simulink model "fftw_15_2_18_18"  (Simulink 7.9)
settings: Solver=ode45  StartTime=0.0  StopTime=10.0  RelTol=1e-3
libraries: xbsIndex_r4 x2224, casper_library_misc x104, casper_library_delays x17, xbsReplacements_r4 x2, casper_library_ffts_twiddle x1, casper_library_reorder x1
(part 19 of 37)

== system fftw_15_2_18_18/fft_wideband_real/fft_direct/butterfly2_1/twiddle_general_4mult/coeff_gen ==
  rst : Inport
  Counter : xbsIndex_r4/Counter  cnt_type=Free Running  cnt_to=Inf  operation=Up  start_count=0  cnt_by_val=1  arith_type=Unsigned  n_bits=13  bin_pt=0  load_pin=off  rst=on  en=off  explicit_period=on  period=1  dbl_ovrd=off  use_behavioral_HDL=off  implementation=Fabric  block_type=counter
  ROM : xbsIndex_r4/ROM  depth=8192  initVector="[0                    -0.0001983642578125                    -0.0003814697265625                     -0.000579833984375                     -0.000762939453125                    -0.0009613037109375                    -0.0011444091796875                       -0.0013427734375                    -0.0015411376953125                    -0.0017242431640625                     -0.001922607421875                     -0.002105712890625                    -0.0023040771484375                    -0.0024871826171875                        -0.002685546875                      -0.00286865234375                    -0.0030670166015625                     -0.003265380859375                     -0.003448486328125                    -0.0036468505859375                    -0.0038299560546875                       -0.0040283203125                      -0.00421142578125                    -0.0044097900390625                     -0.004608154296875                     -0.004791259765625                    -0.0049896240234375                    -0.0051727294921875                         -0.00537109375                      -0.00555419921875                    -0.0057525634765625                     -0.005950927734375                     -0.006134033203125                    -0.0063323974609375                    -0.0065155029296875                       -0.0067138671875                      -0.00689697265625                    -0.0070953369140625                     -0.007293701171875                     -0.007476806640625                    -0.0076751708984375                    -0.0078582763671875                        -0.008056640625                      -0.00823974609375                    -0.0084381103515625                    -0.0086212158203125                     -0.008819580078125                    -0.0090179443359375                    -0.0092010498046875                       -0.0093994140625                      -0.00958251953125                    -0.0097808837890625                    -0.0099639892578125                     -0.010162353515625                    -0.0103607177734375                    -0.0105438232421875                          -0.0107421875                      -0.01092529296875                    -0.0111236572265625                    -0.0113067626953125                     -0.011505126953125                    -0.0117034912109375                    -0.0118865966796875                       -0.0120849609375                      -0.01226806640625                    -0.0124664306640625                    -0.0126495361328125                     -0.012847900390625                     -0.013031005859375                    -0.0132293701171875                        -0.013427734375                      -0.01361083984375                    -0.0138092041015625                    -0.0139923095703125                     -0.014190673828125                     -0.014373779296875                    -0.0145721435546875                       -0.0147705078125                      -0.01495361328125                    -0.0151519775390625                    -0.0153350830078125                     -0.015533447265625                     -0.015716552734375                    -0.0159149169921875                         -0.01611328125                      -0.01629638671875                    -0.0164947509765625                    -0.0166778564453125                     -0.016876220703125                     -0.017059326171875                    -0.0172576904296875                    -0.0174407958984375                      -0.01763916015625                    -0.0178375244140625                    -0.0180206298828125                     -0.018218994140625                     -0.018402099609375                    -0.0186004638671875                    -0.0187835693359375                      -0.01898193359375                    -0.0191802978515625                    -0.0193634033203125                     -0.019561767578125 ...(+315452 chars)]"  distributed_mem=Block RAM  rst=off  init_reg=0  en=off  latency=2  gui_display_data_type=Fixed-point  arith_type="Signed  (2's comp)"  n_bits=18  bin_pt=16  preci_type=Single  exp_width=8  frac_width=24  dbl_ovrd=off  optimize=Area  use_rpm=on  block_type=sprom
  ROM1 : xbsIndex_r4/ROM  depth=8192  initVector="[-1                                     -1                                     -1                                     -1                                     -1                                     -1                                     -1                                     -1                                     -1                                     -1                                     -1                                     -1                                     -1                                     -1                                     -1                                     -1                                     -1                                     -1                                     -1                                     -1                                     -1                    -0.9999847412109375                    -0.9999847412109375                    -0.9999847412109375                    -0.9999847412109375                    -0.9999847412109375                    -0.9999847412109375                    -0.9999847412109375                    -0.9999847412109375                    -0.9999847412109375                    -0.9999847412109375                    -0.9999847412109375                    -0.9999847412109375                    -0.9999847412109375                    -0.9999847412109375                    -0.9999847412109375                     -0.999969482421875                     -0.999969482421875                     -0.999969482421875                     -0.999969482421875                     -0.999969482421875                     -0.999969482421875                     -0.999969482421875                     -0.999969482421875                     -0.999969482421875                     -0.999969482421875                    -0.9999542236328125                    -0.9999542236328125                    -0.9999542236328125                    -0.9999542236328125                    -0.9999542236328125                    -0.9999542236328125                    -0.9999542236328125                    -0.9999542236328125                      -0.99993896484375                      -0.99993896484375                      -0.99993896484375                      -0.99993896484375                      -0.99993896484375                      -0.99993896484375                      -0.99993896484375                      -0.99993896484375                    -0.9999237060546875                    -0.9999237060546875                    -0.9999237060546875                    -0.9999237060546875                    -0.9999237060546875                    -0.9999237060546875                     -0.999908447265625                     -0.999908447265625                     -0.999908447265625                     -0.999908447265625                     -0.999908447265625                     -0.999908447265625                    -0.9998931884765625                    -0.9998931884765625                    -0.9998931884765625                    -0.9998931884765625                    -0.9998931884765625                       -0.9998779296875                       -0.9998779296875                       -0.9998779296875                       -0.9998779296875                       -0.9998779296875                    -0.9998626708984375                    -0.9998626708984375                    -0.9998626708984375                    -0.9998626708984375                    -0.9998626708984375                     -0.999847412109375                     -0.999847412109375                     -0.999847412109375                     -0.999847412109375                     -0.999847412109375                    -0.9998321533203125                    -0.9998321533203125                    -0.9998321533203125                    -0.9998321533203125                      -0.99981689453125                      -0.99981689453125                      -0.99981689453125                      -0.99981689453125                    -0.9998016357421875 ...(+315453 chars)]"  distributed_mem=Block RAM  rst=off  init_reg=0  en=off  latency=2  gui_display_data_type=Fixed-point  arith_type="Signed  (2's comp)"  n_bits=18  bin_pt=16  preci_type=Single  exp_width=8  frac_width=24  dbl_ovrd=off  optimize=Area  use_rpm=on  block_type=sprom
  Slice : xbsIndex_r4/Slice  nbits=13  boolean_output=off  mode=Upper Bit Location + Width  bit1=0  base1=MSB of Input  bit0=0  base0=LSB of Input  dbl_ovrd=off  block_type=slice
  ri_to_c : casper_library_misc/ri_to_c
  w : Outport
  wires:
  ri_to_c/1 -> w/1
  rst/1 -> Counter/1
  Counter/1 -> Slice/1
  Slice/1 -> ROM/1, ROM1/1
  ROM/1 -> ri_to_c/1
  ROM1/1 -> ri_to_c/2
